simulink model "benchmark_no_taylor"  (Simulink 8.8)
settings: Solver=ode45  StartTime=0.0  StopTime=1000  RelTol=1e-3  EnableMultiTasking=on
undefined symbols (not defined in the file or in its folder): sigbuilder_block
libraries: simulink x11, libturbines x10
(part 2 of 3)

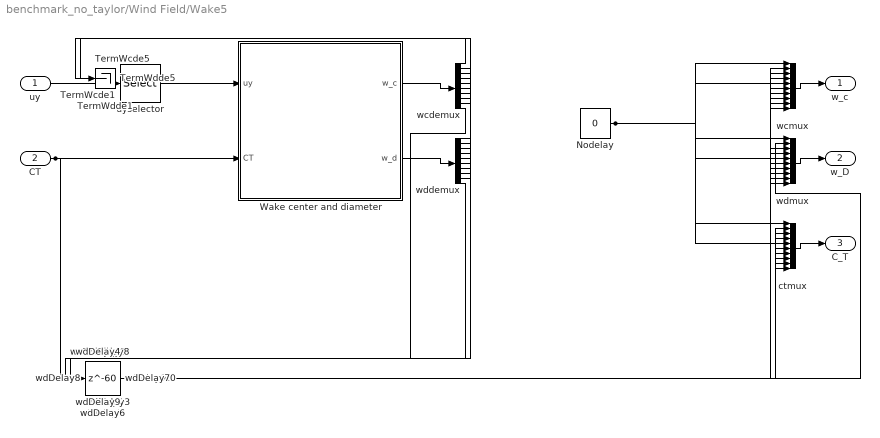
== system benchmark_no_taylor/Wind Field/Wake5 ==
  uy : Inport
  CT : Inport  Port=2
  Nodelay : Constant  Value=0
  TermWcde1 : Terminator
  TermWcde5 : Terminator
  TermWdde1 : Terminator
  TermWdde5 : Terminator
  Wake center and diameter : MATLAB Function  TreatAsAtomicUnit=on
  ctDelay10 : Delay  DelayLength=100  InitialCondition=0  InputProcessing=Inherited  SampleTime=1
  ctDelay2 : Delay  DelayLength=20  InitialCondition=0  InputProcessing=Inherited  SampleTime=1
  ctDelay3 : Delay  DelayLength=60  InitialCondition=0  InputProcessing=Inherited  SampleTime=1
  ctDelay4 : Delay  DelayLength=100  InitialCondition=0  InputProcessing=Inherited  SampleTime=1
  ctDelay6 : Delay  DelayLength=40  InitialCondition=0  InputProcessing=Inherited  SampleTime=1
  ctDelay7 : Delay  DelayLength=80  InitialCondition=0  InputProcessing=Inherited  SampleTime=1
  ctDelay8 : Delay  DelayLength=20  InitialCondition=0  InputProcessing=Inherited  SampleTime=1
  ctDelay9 : Delay  DelayLength=60  InitialCondition=0  InputProcessing=Inherited  SampleTime=1
  ctmux : Mux  Inputs=10
  uyselector : Selector  NumberOfDimensions=2  InputPortWidth=3  IndexOptions=Select all,Index vector (dialog)  Indices=[1 3],p.wt.num  OutputSizes=1,1
  wcDelay10 : Delay  DelayLength=100  InitialCondition=0  InputProcessing=Inherited  SampleTime=1
  wcDelay2 : Delay  DelayLength=20  InitialCondition=0  InputProcessing=Inherited  SampleTime=1
  wcDelay3 : Delay  DelayLength=60  InitialCondition=0  InputProcessing=Inherited  SampleTime=1
  wcDelay4 : Delay  DelayLength=100  InitialCondition=0  InputProcessing=Inherited  SampleTime=1
  wcDelay6 : Delay  DelayLength=40  InitialCondition=0  InputProcessing=Inherited  SampleTime=1
  wcDelay7 : Delay  DelayLength=80  InitialCondition=0  InputProcessing=Inherited  SampleTime=1
  wcDelay8 : Delay  DelayLength=20  InitialCondition=0  InputProcessing=Inherited  SampleTime=1
  wcDelay9 : Delay  DelayLength=60  InitialCondition=0  InputProcessing=Inherited  SampleTime=1
  wcdemux : Demux  Outputs=10
  wcmux : Mux  Inputs=10
  wdDelay10 : Delay  DelayLength=100  InitialCondition=0  InputProcessing=Inherited  SampleTime=1
  wdDelay2 : Delay  DelayLength=20  InitialCondition=0  InputProcessing=Inherited  SampleTime=1
  wdDelay3 : Delay  DelayLength=60  InitialCondition=0  InputProcessing=Inherited  SampleTime=1
  wdDelay4 : Delay  DelayLength=100  InitialCondition=0  InputProcessing=Inherited  SampleTime=1
  wdDelay6 : Delay  DelayLength=40  InitialCondition=0  InputProcessing=Inherited  SampleTime=1
  wdDelay7 : Delay  DelayLength=80  InitialCondition=0  InputProcessing=Inherited  SampleTime=1
  wdDelay8 : Delay  DelayLength=20  InitialCondition=0  InputProcessing=Inherited  SampleTime=1
  wdDelay9 : Delay  DelayLength=60  InitialCondition=0  InputProcessing=Inherited  SampleTime=1
  wddemux : Demux  Outputs=10
  wdmux : Mux  Inputs=10
  w_c : Outport
  w_D : Outport  Port=2
  C_T : Outport  Port=3
  wires:
  ctmux/1 -> C_T/1
  CT/1 -> Wake center and diameter/2(CT), ctDelay2/1, ctDelay3/1, ctDelay4/1, ctDelay6/1, ctDelay7/1, ctDelay8/1, ctDelay9/1, ctDelay10/1
  uy/1 -> uyselector/1
  uyselector/1 -> Wake center and diameter/1(uy)
  Wake center and diameter/1(w_c) -> wcdemux/1
  Wake center and diameter/2(w_d) -> wddemux/1
  wcmux/1 -> w_c/1
  wdmux/1 -> w_D/1
  wddemux/1 -> TermWdde1/1
  wcdemux/1 -> TermWcde1/1
  Nodelay/1 -> wdmux/1, wcmux/1, ctmux/1, wdmux/5, wcmux/5, ctmux/5
  wddemux/2 -> wdDelay2/1
  wdDelay2/1 -> wdmux/2
  wcdemux/2 -> wcDelay2/1
  wcDelay2/1 -> wcmux/2
  ctDelay2/1 -> ctmux/2
  wddemux/3 -> wdDelay3/1
  wdDelay3/1 -> wdmux/3
  wcdemux/3 -> wcDelay3/1
  wcDelay3/1 -> wcmux/3
  ctDelay3/1 -> ctmux/3
  wddemux/4 -> wdDelay4/1
  wdDelay4/1 -> wdmux/4
  wcdemux/4 -> wcDelay4/1
  wcDelay4/1 -> wcmux/4
  ctDelay4/1 -> ctmux/4
  wddemux/5 -> TermWdde5/1
  wcdemux/5 -> TermWcde5/1
  wddemux/6 -> wdDelay6/1
  wdDelay6/1 -> wdmux/6
  wcdemux/6 -> wcDelay6/1
  wcDelay6/1 -> wcmux/6
  ctDelay6/1 -> ctmux/6
  wddemux/7 -> wdDelay7/1
  wdDelay7/1 -> wdmux/7
  wcdemux/7 -> wcDelay7/1
  wcDelay7/1 -> wcmux/7
  ctDelay7/1 -> ctmux/7
  wddemux/8 -> wdDelay8/1
  wdDelay8/1 -> wdmux/8
  wcdemux/8 -> wcDelay8/1
  wcDelay8/1 -> wcmux/8
  ctDelay8/1 -> ctmux/8
  wddemux/9 -> wdDelay9/1
  wdDelay9/1 -> wdmux/9
  wcdemux/9 -> wcDelay9/1
  wcDelay9/1 -> wcmux/9
  ctDelay9/1 -> ctmux/9
  wddemux/10 -> wdDelay10/1
  wdDelay10/1 -> wdmux/10
  wcdemux/10 -> wcDelay10/1
  wcDelay10/1 -> wcmux/10
  ctDelay10/1 -> ctmux/10

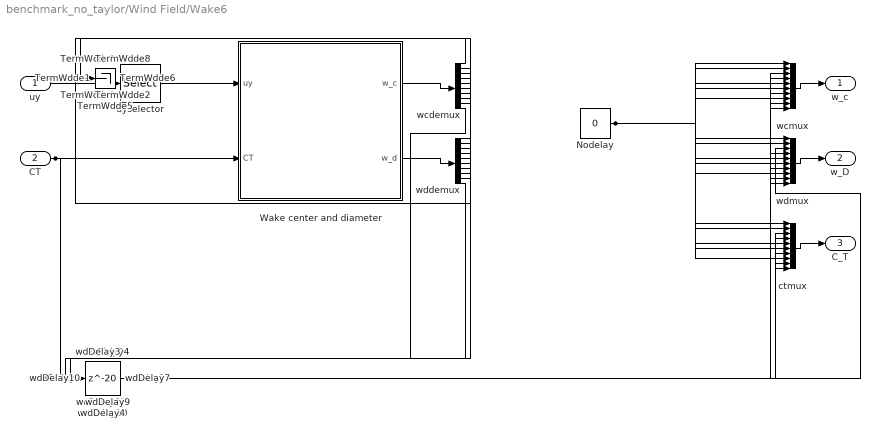
== system benchmark_no_taylor/Wind Field/Wake6 ==
  uy : Inport
  CT : Inport  Port=2
  Nodelay : Constant  Value=0
  TermWcde{1..2,5..6,8} (5 alike) : Terminator
  TermWdde{1..2,5..6,8} (5 alike) : Terminator
  Wake center and diameter : MATLAB Function  TreatAsAtomicUnit=on
  ctDelay10 : Delay  DelayLength=60  InitialCondition=0  InputProcessing=Inherited  SampleTime=1
  ctDelay3 : Delay  DelayLength=20  InitialCondition=0  InputProcessing=Inherited  SampleTime=1
  ctDelay4 : Delay  DelayLength=60  InitialCondition=0  InputProcessing=Inherited  SampleTime=1
  ctDelay7 : Delay  DelayLength=40  InitialCondition=0  InputProcessing=Inherited  SampleTime=1
  ctDelay9 : Delay  DelayLength=20  InitialCondition=0  InputProcessing=Inherited  SampleTime=1
  ctmux : Mux  Inputs=10
  uyselector : Selector  NumberOfDimensions=2  InputPortWidth=3  IndexOptions=Select all,Index vector (dialog)  Indices=[1 3],p.wt.num  OutputSizes=1,1
  wcDelay10 : Delay  DelayLength=60  InitialCondition=0  InputProcessing=Inherited  SampleTime=1
  wcDelay3 : Delay  DelayLength=20  InitialCondition=0  InputProcessing=Inherited  SampleTime=1
  wcDelay4 : Delay  DelayLength=60  InitialCondition=0  InputProcessing=Inherited  SampleTime=1
  wcDelay7 : Delay  DelayLength=40  InitialCondition=0  InputProcessing=Inherited  SampleTime=1
  wcDelay9 : Delay  DelayLength=20  InitialCondition=0  InputProcessing=Inherited  SampleTime=1
  wcdemux : Demux  Outputs=10
  wcmux : Mux  Inputs=10
  wdDelay10 : Delay  DelayLength=60  InitialCondition=0  InputProcessing=Inherited  SampleTime=1
  wdDelay3 : Delay  DelayLength=20  InitialCondition=0  InputProcessing=Inherited  SampleTime=1
  wdDelay4 : Delay  DelayLength=60  InitialCondition=0  InputProcessing=Inherited  SampleTime=1
  wdDelay7 : Delay  DelayLength=40  InitialCondition=0  InputProcessing=Inherited  SampleTime=1
  wdDelay9 : Delay  DelayLength=20  InitialCondition=0  InputProcessing=Inherited  SampleTime=1
  wddemux : Demux  Outputs=10
  wdmux : Mux  Inputs=10
  w_c : Outport
  w_D : Outport  Port=2
  C_T : Outport  Port=3
  wires:
  ctmux/1 -> C_T/1
  CT/1 -> Wake center and diameter/2(CT), ctDelay3/1, ctDelay4/1, ctDelay7/1, ctDelay9/1, ctDelay10/1
  uy/1 -> uyselector/1
  uyselector/1 -> Wake center and diameter/1(uy)
  Wake center and diameter/1(w_c) -> wcdemux/1
  Wake center and diameter/2(w_d) -> wddemux/1
  wcmux/1 -> w_c/1
  wdmux/1 -> w_D/1
  wddemux/1 -> TermWdde1/1
  wcdemux/1 -> TermWcde1/1
  Nodelay/1 -> wdmux/1, wcmux/1, ctmux/1, wdmux/2, wcmux/2, ctmux/2, wdmux/5, wcmux/5, ctmux/5, wdmux/6, wcmux/6, ctmux/6, wdmux/8, wcmux/8, ctmux/8
  wddemux/2 -> TermWdde2/1
  wcdemux/2 -> TermWcde2/1
  wddemux/3 -> wdDelay3/1
  wdDelay3/1 -> wdmux/3
  wcdemux/3 -> wcDelay3/1
  wcDelay3/1 -> wcmux/3
  ctDelay3/1 -> ctmux/3
  wddemux/4 -> wdDelay4/1
  wdDelay4/1 -> wdmux/4
  wcdemux/4 -> wcDelay4/1
  wcDelay4/1 -> wcmux/4
  ctDelay4/1 -> ctmux/4
  wddemux/5 -> TermWdde5/1
  wcdemux/5 -> TermWcde5/1
  wddemux/6 -> TermWdde6/1
  wcdemux/6 -> TermWcde6/1
  wddemux/7 -> wdDelay7/1
  wdDelay7/1 -> wdmux/7
  wcdemux/7 -> wcDelay7/1
  wcDelay7/1 -> wcmux/7
  ctDelay7/1 -> ctmux/7
  wddemux/8 -> TermWdde8/1
  wcdemux/8 -> TermWcde8/1
  wddemux/9 -> wdDelay9/1
  wdDelay9/1 -> wdmux/9
  wcdemux/9 -> wcDelay9/1
  wcDelay9/1 -> wcmux/9
  ctDelay9/1 -> ctmux/9
  wddemux/10 -> wdDelay10/1
  wdDelay10/1 -> wdmux/10
  wcdemux/10 -> wcDelay10/1
  wcDelay10/1 -> wcmux/10
  ctDelay10/1 -> ctmux/10

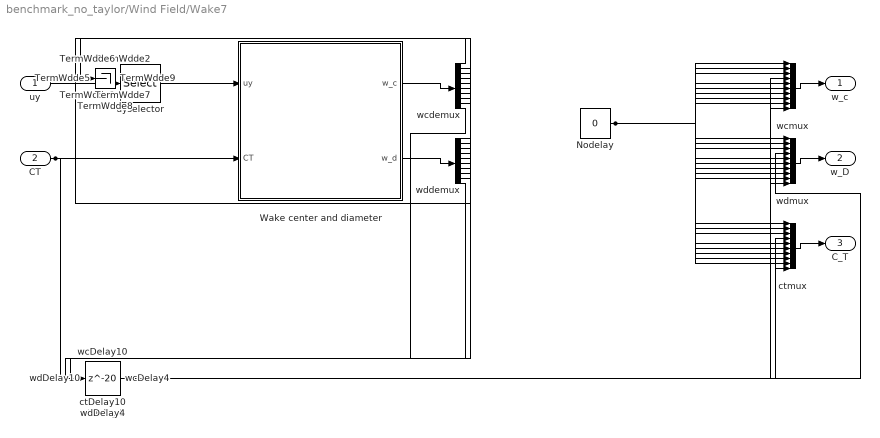
== system benchmark_no_taylor/Wind Field/Wake7 ==
  uy : Inport
  CT : Inport  Port=2
  Nodelay : Constant  Value=0
  TermWcde{1..3,5..9} (8 alike) : Terminator
  TermWdde{1..3,5..9} (8 alike) : Terminator
  Wake center and diameter : MATLAB Function  TreatAsAtomicUnit=on
  ctDelay10 : Delay  DelayLength=20  InitialCondition=0  InputProcessing=Inherited  SampleTime=1
  ctDelay4 : Delay  DelayLength=20  InitialCondition=0  InputProcessing=Inherited  SampleTime=1
  ctmux : Mux  Inputs=10
  uyselector : Selector  NumberOfDimensions=2  InputPortWidth=3  IndexOptions=Select all,Index vector (dialog)  Indices=[1 3],p.wt.num  OutputSizes=1,1
  wcDelay10 : Delay  DelayLength=20  InitialCondition=0  InputProcessing=Inherited  SampleTime=1
  wcDelay4 : Delay  DelayLength=20  InitialCondition=0  InputProcessing=Inherited  SampleTime=1
  wcdemux : Demux  Outputs=10
  wcmux : Mux  Inputs=10
  wdDelay10 : Delay  DelayLength=20  InitialCondition=0  InputProcessing=Inherited  SampleTime=1
  wdDelay4 : Delay  DelayLength=20  InitialCondition=0  InputProcessing=Inherited  SampleTime=1
  wddemux : Demux  Outputs=10
  wdmux : Mux  Inputs=10
  w_c : Outport
  w_D : Outport  Port=2
  C_T : Outport  Port=3
  wires:
  ctmux/1 -> C_T/1
  CT/1 -> Wake center and diameter/2(CT), ctDelay4/1, ctDelay10/1
  uy/1 -> uyselector/1
  uyselector/1 -> Wake center and diameter/1(uy)
  Wake center and diameter/1(w_c) -> wcdemux/1
  Wake center and diameter/2(w_d) -> wddemux/1
  wcmux/1 -> w_c/1
  wdmux/1 -> w_D/1
  wddemux/1 -> TermWdde1/1
  wcdemux/1 -> TermWcde1/1
  Nodelay/1 -> wdmux/1, wcmux/1, ctmux/1, wdmux/2, wcmux/2, ctmux/2, wdmux/3, wcmux/3, ctmux/3, wdmux/5, wcmux/5, ctmux/5, wdmux/6, wcmux/6, ctmux/6, wdmux/7, wcmux/7, ctmux/7, wdmux/8, wcmux/8, ctmux/8, wdmux/9, wcmux/9, ctmux/9
  wddemux/2 -> TermWdde2/1
  wcdemux/2 -> TermWcde2/1
  wddemux/3 -> TermWdde3/1
  wcdemux/3 -> TermWcde3/1
  wddemux/4 -> wdDelay4/1
  wdDelay4/1 -> wdmux/4
  wcdemux/4 -> wcDelay4/1
  wcDelay4/1 -> wcmux/4
  ctDelay4/1 -> ctmux/4
  wddemux/5 -> TermWdde5/1
  wcdemux/5 -> TermWcde5/1
  wddemux/6 -> TermWdde6/1
  wcdemux/6 -> TermWcde6/1
  wddemux/7 -> TermWdde7/1
  wcdemux/7 -> TermWcde7/1
  wddemux/8 -> TermWdde8/1
  wcdemux/8 -> TermWcde8/1
  wddemux/9 -> TermWdde9/1
  wcdemux/9 -> TermWcde9/1
  wddemux/10 -> wdDelay10/1
  wdDelay10/1 -> wdmux/10
  wcdemux/10 -> wcDelay10/1
  wcDelay10/1 -> wcmux/10
  ctDelay10/1 -> ctmux/10

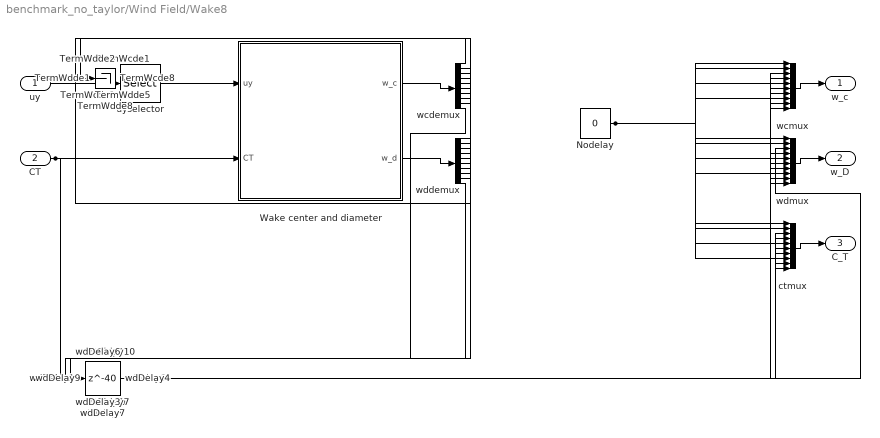
== system benchmark_no_taylor/Wind Field/Wake8 ==
  uy : Inport
  CT : Inport  Port=2
  Nodelay : Constant  Value=0
  TermWcde{1..2,5,8} (4 alike) : Terminator
  TermWdde{1..2,5,8} (4 alike) : Terminator
  Wake center and diameter : MATLAB Function  TreatAsAtomicUnit=on
  ctDelay10 : Delay  DelayLength=80  InitialCondition=0  InputProcessing=Inherited  SampleTime=1
  ctDelay3 : Delay  DelayLength=40  InitialCondition=0  InputProcessing=Inherited  SampleTime=1
  ctDelay4 : Delay  DelayLength=80  InitialCondition=0  InputProcessing=Inherited  SampleTime=1
  ctDelay6 : Delay  DelayLength=20  InitialCondition=0  InputProcessing=Inherited  SampleTime=1
  ctDelay7 : Delay  DelayLength=60  InitialCondition=0  InputProcessing=Inherited  SampleTime=1
  ctDelay9 : Delay  DelayLength=40  InitialCondition=0  InputProcessing=Inherited  SampleTime=1
  ctmux : Mux  Inputs=10
  uyselector : Selector  NumberOfDimensions=2  InputPortWidth=3  IndexOptions=Select all,Index vector (dialog)  Indices=[1 3],p.wt.num  OutputSizes=1,1
  wcDelay10 : Delay  DelayLength=80  InitialCondition=0  InputProcessing=Inherited  SampleTime=1
  wcDelay3 : Delay  DelayLength=40  InitialCondition=0  InputProcessing=Inherited  SampleTime=1
  wcDelay4 : Delay  DelayLength=80  InitialCondition=0  InputProcessing=Inherited  SampleTime=1
  wcDelay6 : Delay  DelayLength=20  InitialCondition=0  InputProcessing=Inherited  SampleTime=1
  wcDelay7 : Delay  DelayLength=60  InitialCondition=0  InputProcessing=Inherited  SampleTime=1
  wcDelay9 : Delay  DelayLength=40  InitialCondition=0  InputProcessing=Inherited  SampleTime=1
  wcdemux : Demux  Outputs=10
  wcmux : Mux  Inputs=10
  wdDelay10 : Delay  DelayLength=80  InitialCondition=0  InputProcessing=Inherited  SampleTime=1
  wdDelay3 : Delay  DelayLength=40  InitialCondition=0  InputProcessing=Inherited  SampleTime=1
  wdDelay4 : Delay  DelayLength=80  InitialCondition=0  InputProcessing=Inherited  SampleTime=1
  wdDelay6 : Delay  DelayLength=20  InitialCondition=0  InputProcessing=Inherited  SampleTime=1
  wdDelay7 : Delay  DelayLength=60  InitialCondition=0  InputProcessing=Inherited  SampleTime=1
  wdDelay9 : Delay  DelayLength=40  InitialCondition=0  InputProcessing=Inherited  SampleTime=1
  wddemux : Demux  Outputs=10
  wdmux : Mux  Inputs=10
  w_c : Outport
  w_D : Outport  Port=2
  C_T : Outport  Port=3
  wires:
  ctmux/1 -> C_T/1
  CT/1 -> Wake center and diameter/2(CT), ctDelay3/1, ctDelay4/1, ctDelay6/1, ctDelay7/1, ctDelay9/1, ctDelay10/1
  uy/1 -> uyselector/1
  uyselector/1 -> Wake center and diameter/1(uy)
  Wake center and diameter/1(w_c) -> wcdemux/1
  Wake center and diameter/2(w_d) -> wddemux/1
  wcmux/1 -> w_c/1
  wdmux/1 -> w_D/1
  wddemux/1 -> TermWdde1/1
  wcdemux/1 -> TermWcde1/1
  Nodelay/1 -> wdmux/1, wcmux/1, ctmux/1, wdmux/2, wcmux/2, ctmux/2, wdmux/5, wcmux/5, ctmux/5, wdmux/8, wcmux/8, ctmux/8
  wddemux/2 -> TermWdde2/1
  wcdemux/2 -> TermWcde2/1
  wddemux/3 -> wdDelay3/1
  wdDelay3/1 -> wdmux/3
  wcdemux/3 -> wcDelay3/1
  wcDelay3/1 -> wcmux/3
  ctDelay3/1 -> ctmux/3
  wddemux/4 -> wdDelay4/1
  wdDelay4/1 -> wdmux/4
  wcdemux/4 -> wcDelay4/1
  wcDelay4/1 -> wcmux/4
  ctDelay4/1 -> ctmux/4
  wddemux/5 -> TermWdde5/1
  wcdemux/5 -> TermWcde5/1
  wddemux/6 -> wdDelay6/1
  wdDelay6/1 -> wdmux/6
  wcdemux/6 -> wcDelay6/1
  wcDelay6/1 -> wcmux/6
  ctDelay6/1 -> ctmux/6
  wddemux/7 -> wdDelay7/1
  wdDelay7/1 -> wdmux/7
  wcdemux/7 -> wcDelay7/1
  wcDelay7/1 -> wcmux/7
  ctDelay7/1 -> ctmux/7
  wddemux/8 -> TermWdde8/1
  wcdemux/8 -> TermWcde8/1
  wddemux/9 -> wdDelay9/1
  wdDelay9/1 -> wdmux/9
  wcdemux/9 -> wcDelay9/1
  wcDelay9/1 -> wcmux/9
  ctDelay9/1 -> ctmux/9
  wddemux/10 -> wdDelay10/1
  wdDelay10/1 -> wdmux/10
  wcdemux/10 -> wcDelay10/1
  wcDelay10/1 -> wcmux/10
  ctDelay10/1 -> ctmux/10

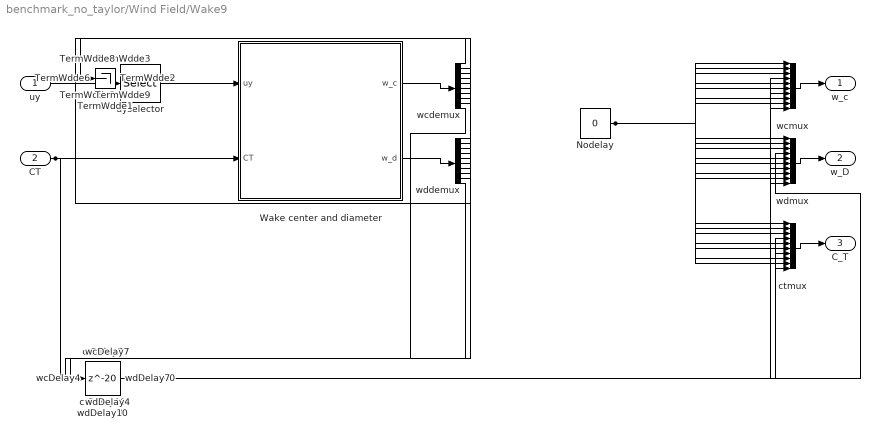
== system benchmark_no_taylor/Wind Field/Wake9 ==
  uy : Inport
  CT : Inport  Port=2
  Nodelay : Constant  Value=0
  TermWcde{1..3,5..6,8..9} (7 alike) : Terminator
  TermWdde{1..3,5..6,8..9} (7 alike) : Terminator
  Wake center and diameter : MATLAB Function  TreatAsAtomicUnit=on
  ctDelay10 : Delay  DelayLength=40  InitialCondition=0  InputProcessing=Inherited  SampleTime=1
  ctDelay4 : Delay  DelayLength=40  InitialCondition=0  InputProcessing=Inherited  SampleTime=1
  ctDelay7 : Delay  DelayLength=20  InitialCondition=0  InputProcessing=Inherited  SampleTime=1
  ctmux : Mux  Inputs=10
  uyselector : Selector  NumberOfDimensions=2  InputPortWidth=3  IndexOptions=Select all,Index vector (dialog)  Indices=[1 3],p.wt.num  OutputSizes=1,1
  wcDelay10 : Delay  DelayLength=40  InitialCondition=0  InputProcessing=Inherited  SampleTime=1
  wcDelay4 : Delay  DelayLength=40  InitialCondition=0  InputProcessing=Inherited  SampleTime=1
  wcDelay7 : Delay  DelayLength=20  InitialCondition=0  InputProcessing=Inherited  SampleTime=1
  wcdemux : Demux  Outputs=10
  wcmux : Mux  Inputs=10
  wdDelay10 : Delay  DelayLength=40  InitialCondition=0  InputProcessing=Inherited  SampleTime=1
  wdDelay4 : Delay  DelayLength=40  InitialCondition=0  InputProcessing=Inherited  SampleTime=1
  wdDelay7 : Delay  DelayLength=20  InitialCondition=0  InputProcessing=Inherited  SampleTime=1
  wddemux : Demux  Outputs=10
  wdmux : Mux  Inputs=10
  w_c : Outport
  w_D : Outport  Port=2
  C_T : Outport  Port=3
  wires:
  ctmux/1 -> C_T/1
  CT/1 -> Wake center and diameter/2(CT), ctDelay4/1, ctDelay7/1, ctDelay10/1
  uy/1 -> uyselector/1
  uyselector/1 -> Wake center and diameter/1(uy)
  Wake center and diameter/1(w_c) -> wcdemux/1
  Wake center and diameter/2(w_d) -> wddemux/1
  wcmux/1 -> w_c/1
  wdmux/1 -> w_D/1
  wddemux/1 -> TermWdde1/1
  wcdemux/1 -> TermWcde1/1
  Nodelay/1 -> wdmux/1, wcmux/1, ctmux/1, wdmux/2, wcmux/2, ctmux/2, wdmux/3, wcmux/3, ctmux/3, wdmux/5, wcmux/5, ctmux/5, wdmux/6, wcmux/6, ctmux/6, wdmux/8, wcmux/8, ctmux/8, wdmux/9, wcmux/9, ctmux/9
  wddemux/2 -> TermWdde2/1
  wcdemux/2 -> TermWcde2/1
  wddemux/3 -> TermWdde3/1
  wcdemux/3 -> TermWcde3/1
  wddemux/4 -> wdDelay4/1
  wdDelay4/1 -> wdmux/4
  wcdemux/4 -> wcDelay4/1
  wcDelay4/1 -> wcmux/4
  ctDelay4/1 -> ctmux/4
  wddemux/5 -> TermWdde5/1
  wcdemux/5 -> TermWcde5/1
  wddemux/6 -> TermWdde6/1
  wcdemux/6 -> TermWcde6/1
  wddemux/7 -> wdDelay7/1
  wdDelay7/1 -> wdmux/7
  wcdemux/7 -> wcDelay7/1
  wcDelay7/1 -> wcmux/7
  ctDelay7/1 -> ctmux/7
  wddemux/8 -> TermWdde8/1
  wcdemux/8 -> TermWcde8/1
  wddemux/9 -> TermWdde9/1
  wcdemux/9 -> TermWcde9/1
  wddemux/10 -> wdDelay10/1
  wdDelay10/1 -> wdmux/10
  wcdemux/10 -> wcDelay10/1
  wcDelay10/1 -> wcmux/10
  ctDelay10/1 -> ctmux/10

== system benchmark_no_taylor/Wind Field/Wind Speed1 ==
  def : Inport
  Counter Free-Running : simulink/Sources/Counter Free-Running  NumBits=32  tsamp=p.ts
  Selector1 : Selector  NumberOfDimensions=2  IndexMode=Zero-based  InputPortWidth=3  IndexOptions=Select all,Index vector (port)  Indices=[1 3],1  OutputSizes=1,1
  Signal Conversion : SignalConversion  ConversionOutput=Contiguous copy  OverrideOpt=off
  Signal Conversion1 : SignalConversion  ConversionOutput=Contiguous copy  OverrideOpt=off
  Ux_full : Constant  Value=p.wind'
  Wind speed : MATLAB Function  TreatAsAtomicUnit=on
  v_rot : Outport
  v_nac : Outport  Port=2
  wires:
  def/1 -> Wind speed/2(def)
  Wind speed/2(upoint) -> Signal Conversion1/1
  Wind speed/1(uavg) -> Signal Conversion/1
  Counter Free-Running/1 -> Selector1/2
  Ux_full/1 -> Selector1/1
  Selector1/1 -> Wind speed/1(ux)
  Signal Conversion/1 -> v_rot/1
  Signal Conversion1/1 -> v_nac/1

== system benchmark_no_taylor/Wind Field/Wind Speed10 ==
  def : Inport
  Counter Free-Running : simulink/Sources/Counter Free-Running  NumBits=32  tsamp=p.ts
  Selector1 : Selector  NumberOfDimensions=2  IndexMode=Zero-based  InputPortWidth=3  IndexOptions=Select all,Index vector (port)  Indices=[1 3],1  OutputSizes=1,1
  Signal Conversion : SignalConversion  ConversionOutput=Contiguous copy  OverrideOpt=off
  Signal Conversion1 : SignalConversion  ConversionOutput=Contiguous copy  OverrideOpt=off
  Ux_full : Constant  Value=p.wind'
  Wind speed : MATLAB Function  TreatAsAtomicUnit=on
  v_rot : Outport
  v_nac : Outport  Port=2
  wires:
  def/1 -> Wind speed/2(def)
  Wind speed/2(upoint) -> Signal Conversion1/1
  Wind speed/1(uavg) -> Signal Conversion/1
  Counter Free-Running/1 -> Selector1/2
  Ux_full/1 -> Selector1/1
  Selector1/1 -> Wind speed/1(ux)
  Signal Conversion/1 -> v_rot/1
  Signal Conversion1/1 -> v_nac/1

== system benchmark_no_taylor/Wind Field/Wind Speed2 ==
  def : Inport
  Counter Free-Running : simulink/Sources/Counter Free-Running  NumBits=32  tsamp=p.ts
  Selector1 : Selector  NumberOfDimensions=2  IndexMode=Zero-based  InputPortWidth=3  IndexOptions=Select all,Index vector (port)  Indices=[1 3],1  OutputSizes=1,1
  Signal Conversion : SignalConversion  ConversionOutput=Contiguous copy  OverrideOpt=off
  Signal Conversion1 : SignalConversion  ConversionOutput=Contiguous copy  OverrideOpt=off
  Ux_full : Constant  Value=p.wind'
  Wind speed : MATLAB Function  TreatAsAtomicUnit=on
  v_rot : Outport
  v_nac : Outport  Port=2
  wires:
  def/1 -> Wind speed/2(def)
  Wind speed/2(upoint) -> Signal Conversion1/1
  Wind speed/1(uavg) -> Signal Conversion/1
  Counter Free-Running/1 -> Selector1/2
  Ux_full/1 -> Selector1/1
  Selector1/1 -> Wind speed/1(ux)
  Signal Conversion/1 -> v_rot/1
  Signal Conversion1/1 -> v_nac/1

== system benchmark_no_taylor/Wind Field/Wind Speed3 ==
  def : Inport
  Counter Free-Running : simulink/Sources/Counter Free-Running  NumBits=32  tsamp=p.ts
  Selector1 : Selector  NumberOfDimensions=2  IndexMode=Zero-based  InputPortWidth=3  IndexOptions=Select all,Index vector (port)  Indices=[1 3],1  OutputSizes=1,1
  Signal Conversion : SignalConversion  ConversionOutput=Contiguous copy  OverrideOpt=off
  Signal Conversion1 : SignalConversion  ConversionOutput=Contiguous copy  OverrideOpt=off
  Ux_full : Constant  Value=p.wind'
  Wind speed : MATLAB Function  TreatAsAtomicUnit=on
  v_rot : Outport
  v_nac : Outport  Port=2
  wires:
  def/1 -> Wind speed/2(def)
  Wind speed/2(upoint) -> Signal Conversion1/1
  Wind speed/1(uavg) -> Signal Conversion/1
  Counter Free-Running/1 -> Selector1/2
  Ux_full/1 -> Selector1/1
  Selector1/1 -> Wind speed/1(ux)
  Signal Conversion/1 -> v_rot/1
  Signal Conversion1/1 -> v_nac/1

== system benchmark_no_taylor/Wind Field/Wind Speed4 ==
  def : Inport
  Counter Free-Running : simulink/Sources/Counter Free-Running  NumBits=32  tsamp=p.ts
  Selector1 : Selector  NumberOfDimensions=2  IndexMode=Zero-based  InputPortWidth=3  IndexOptions=Select all,Index vector (port)  Indices=[1 3],1  OutputSizes=1,1
  Signal Conversion : SignalConversion  ConversionOutput=Contiguous copy  OverrideOpt=off
  Signal Conversion1 : SignalConversion  ConversionOutput=Contiguous copy  OverrideOpt=off
  Ux_full : Constant  Value=p.wind'
  Wind speed : MATLAB Function  TreatAsAtomicUnit=on
  v_rot : Outport
  v_nac : Outport  Port=2
  wires:
  def/1 -> Wind speed/2(def)
  Wind speed/2(upoint) -> Signal Conversion1/1
  Wind speed/1(uavg) -> Signal Conversion/1
  Counter Free-Running/1 -> Selector1/2
  Ux_full/1 -> Selector1/1
  Selector1/1 -> Wind speed/1(ux)
  Signal Conversion/1 -> v_rot/1
  Signal Conversion1/1 -> v_nac/1

== system benchmark_no_taylor/Wind Field/Wind Speed5 ==
  def : Inport
  Counter Free-Running : simulink/Sources/Counter Free-Running  NumBits=32  tsamp=p.ts
  Selector1 : Selector  NumberOfDimensions=2  IndexMode=Zero-based  InputPortWidth=3  IndexOptions=Select all,Index vector (port)  Indices=[1 3],1  OutputSizes=1,1
  Signal Conversion : SignalConversion  ConversionOutput=Contiguous copy  OverrideOpt=off
  Signal Conversion1 : SignalConversion  ConversionOutput=Contiguous copy  OverrideOpt=off
  Ux_full : Constant  Value=p.wind'
  Wind speed : MATLAB Function  TreatAsAtomicUnit=on
  v_rot : Outport
  v_nac : Outport  Port=2
  wires:
  def/1 -> Wind speed/2(def)
  Wind speed/2(upoint) -> Signal Conversion1/1
  Wind speed/1(uavg) -> Signal Conversion/1
  Counter Free-Running/1 -> Selector1/2
  Ux_full/1 -> Selector1/1
  Selector1/1 -> Wind speed/1(ux)
  Signal Conversion/1 -> v_rot/1
  Signal Conversion1/1 -> v_nac/1

== system benchmark_no_taylor/Wind Field/Wind Speed6 ==
  def : Inport
  Counter Free-Running : simulink/Sources/Counter Free-Running  NumBits=32  tsamp=p.ts
  Selector1 : Selector  NumberOfDimensions=2  IndexMode=Zero-based  InputPortWidth=3  IndexOptions=Select all,Index vector (port)  Indices=[1 3],1  OutputSizes=1,1
  Signal Conversion : SignalConversion  ConversionOutput=Contiguous copy  OverrideOpt=off
  Signal Conversion1 : SignalConversion  ConversionOutput=Contiguous copy  OverrideOpt=off
  Ux_full : Constant  Value=p.wind'
  Wind speed : MATLAB Function  TreatAsAtomicUnit=on
  v_rot : Outport
  v_nac : Outport  Port=2
  wires:
  def/1 -> Wind speed/2(def)
  Wind speed/2(upoint) -> Signal Conversion1/1
  Wind speed/1(uavg) -> Signal Conversion/1
  Counter Free-Running/1 -> Selector1/2
  Ux_full/1 -> Selector1/1
  Selector1/1 -> Wind speed/1(ux)
  Signal Conversion/1 -> v_rot/1
  Signal Conversion1/1 -> v_nac/1

== system benchmark_no_taylor/Wind Field/Wind Speed7 ==
  def : Inport
  Counter Free-Running : simulink/Sources/Counter Free-Running  NumBits=32  tsamp=p.ts
  Selector1 : Selector  NumberOfDimensions=2  IndexMode=Zero-based  InputPortWidth=3  IndexOptions=Select all,Index vector (port)  Indices=[1 3],1  OutputSizes=1,1
  Signal Conversion : SignalConversion  ConversionOutput=Contiguous copy  OverrideOpt=off
  Signal Conversion1 : SignalConversion  ConversionOutput=Contiguous copy  OverrideOpt=off
  Ux_full : Constant  Value=p.wind'
  Wind speed : MATLAB Function  TreatAsAtomicUnit=on
  v_rot : Outport
  v_nac : Outport  Port=2
  wires:
  def/1 -> Wind speed/2(def)
  Wind speed/2(upoint) -> Signal Conversion1/1
  Wind speed/1(uavg) -> Signal Conversion/1
  Counter Free-Running/1 -> Selector1/2
  Ux_full/1 -> Selector1/1
  Selector1/1 -> Wind speed/1(ux)
  Signal Conversion/1 -> v_rot/1
  Signal Conversion1/1 -> v_nac/1

== system benchmark_no_taylor/Wind Field/Wind Speed8 ==
  def : Inport
  Counter Free-Running : simulink/Sources/Counter Free-Running  NumBits=32  tsamp=p.ts
  Selector1 : Selector  NumberOfDimensions=2  IndexMode=Zero-based  InputPortWidth=3  IndexOptions=Select all,Index vector (port)  Indices=[1 3],1  OutputSizes=1,1
  Signal Conversion : SignalConversion  ConversionOutput=Contiguous copy  OverrideOpt=off
  Signal Conversion1 : SignalConversion  ConversionOutput=Contiguous copy  OverrideOpt=off
  Ux_full : Constant  Value=p.wind'
  Wind speed : MATLAB Function  TreatAsAtomicUnit=on
  v_rot : Outport
  v_nac : Outport  Port=2
  wires:
  def/1 -> Wind speed/2(def)
  Wind speed/2(upoint) -> Signal Conversion1/1
  Wind speed/1(uavg) -> Signal Conversion/1
  Counter Free-Running/1 -> Selector1/2
  Ux_full/1 -> Selector1/1
  Selector1/1 -> Wind speed/1(ux)
  Signal Conversion/1 -> v_rot/1
  Signal Conversion1/1 -> v_nac/1

== system benchmark_no_taylor/Wind Field/Wind Speed9 ==
  def : Inport
  Counter Free-Running : simulink/Sources/Counter Free-Running  NumBits=32  tsamp=p.ts
  Selector1 : Selector  NumberOfDimensions=2  IndexMode=Zero-based  InputPortWidth=3  IndexOptions=Select all,Index vector (port)  Indices=[1 3],1  OutputSizes=1,1
  Signal Conversion : SignalConversion  ConversionOutput=Contiguous copy  OverrideOpt=off
  Signal Conversion1 : SignalConversion  ConversionOutput=Contiguous copy  OverrideOpt=off
  Ux_full : Constant  Value=p.wind'
  Wind speed : MATLAB Function  TreatAsAtomicUnit=on
  v_rot : Outport
  v_nac : Outport  Port=2
  wires:
  def/1 -> Wind speed/2(def)
  Wind speed/2(upoint) -> Signal Conversion1/1
  Wind speed/1(uavg) -> Signal Conversion/1
  Counter Free-Running/1 -> Selector1/2
  Ux_full/1 -> Selector1/1
  Selector1/1 -> Wind speed/1(ux)
  Signal Conversion/1 -> v_rot/1
  Signal Conversion1/1 -> v_nac/1

== matlab function Farm Control/control ==
  data: v_nac input double; P_demand input double; parm parameter double; P_ref output double; P_avail output double
function [P_ref,P_avail]  = Farm_Controller(v_nac,P_demand,parm)
%
% Farm_Controller estimates the available power at the farm and distributes the 
% power references proportional to the available power at each wind turbine.
%
% [P_ref,P_avail_total]  = Farm_Controller(v_nac,P_demand)
%
% vn : the wind speed at wind turbine number n.
%
% P_demand : The demanded power by the operator.
%
% P_ref : The power reference for the wind turbines.
%
% P_avail : Available power at the farm level
%
%
% Version 1: 2009-04-10 sms
% Version 2: 2009-12-04 grunnet
% By : S. Mohsen N. Soltani & Jacob D. Grunnet
% Aalborg University, Dept. of Electronic Systems, Section of Automation
% and Control
% E-mail: sms@es.aau.dk
% E-mail: grunnet@es.aau.dk

rho=parm.rho; %air dencity
R=parm.radius; %rotor radius
rated=parm.rated; %Rated power
N=parm.N; %Number of turbines
Cp=parm.Cp; % Max cp of the turbines

P_a=zeros(N,1);
P_ref=zeros(N,1);

% Compute available power at each turbine
for i=1:N
    %P_a=1/2*pi*r^2*Cp*v^3
    P_a(i)=min(rated(i),(pi/2)*rho*R(i)^2*v_nac(i)^3*Cp(i));
end

%Compute total available power
P_avail=sum(P_a);

%Distribute power according to availibility
for i=1:N
    P_ref(i)=max(0,min(rated(i),P_demand*P_a(i)/P_avail));
end

== matlab function Network Operator/Network Operator ==
  data: P_avail input double; settings input double; f input double; P_demand output double; P_demand0 input double; parm parameter double
function P_demand = TSO(P_avail,settings,f,P_demand0,parm)
%
% TSO provides the demanded power to the wind farm controller.
%
% P_avail : Aavailable power at the farm level.
%
% mode : The operation mode.
%
% settings : The settings corresponding to the operational mode
%
% f : Frequency of the network
%
% P_deamnd0 : The last update of the deamded power
%
% Ts : Sample Time
%
% Time-stamp: <2009-04-10 sms>
% Version 1: 2009-04-10 sms 
% By : S. Mohsen N. Soltani
% Aalborg University, Dept. of Electronic Systems, Section of Automation
% and Control
% E-mail: sms@es.aau.dk
% 
 P=0;
 switch(parm.mode)
     case 1 %Absolute active power control
        P=settings(1);
%       % type='Full power';
     case 2 %Delta mode
        P=P_avail-settings(1);
%       % type='Delta mode';
     case 3 %Balance
        P=settings(1);
%       % type='Balance';
     case 4 %Rate limiter
        P=min(P_avail,settings(1)*parm.Ts+P_demand0);
%       % type='Rate limiter';
     case 5 %Frequency Control
        deadb=settings(1);
        contb=settings(2);
        maxp=settings(3);
        minp=settings(4);
        f=f-settings(5); % To provide Delta f
        if ( f<= deadb && f>=-deadb)
            P=0.5*(maxp+minp);
        elseif f>= contb
            P=minp;
        elseif f<= -contb
            P=maxp;
        elseif ( f>deadb && f<contb)
            P=0.5*(maxp+minp)-0.5*(maxp-minp)*(f-deadb)/(contb-deadb);
        elseif ( f<-deadb && f>-contb)
            P=0.5*(maxp+minp)-0.5*(maxp-minp)*(f+deadb)/(contb-deadb);
        end
%       % type='Frequency Control';
     otherwise %Unknown
        P=P_avail;
%       % type='Unknown Mode';
 end
%        

P_demand=max(0,P);

== matlab function Wind Field/Deficit ==
  data: w_c input double; w_d input double; ct_dw input double; p parameter double; def output double
function def  = deficit(w_c,w_d,ct_dw,p) %#eml
% w_c   is the vector of wake centers from the other turbins at the x position of the turbine
% w_d   is the vector of wake diameters from the other turbins at the x position of the turbine
% ct_dw is the vector of thrust coefficients from the other turbins at the x position of the turbine
% p     is a structure containing farm layout and tubine rotor sizes  

wake_from = zeros(length(p.farm.x),length(p.farm.x));
dist_i = zeros(length(p.farm.x),1);
def = ones(length(p.farm.x),1);
if ct_dw(size(ct_dw,1),1) ~= 0 % only calculate deficit if wind has reached end of farm
    [~, dist_i] = sort(p.farm.x);

    %This for-loop make a "tree" of which turbines are in the wake
    for i=1:length(p.farm.x)
        turb_i = dist_i(i);
        for k = 1:length(p.farm.x)
            turb_k = dist_i(k);
            temp = p.farm.y(turb_k)-w_c(turb_k,turb_i);
            %if turb_k is in turb_i's wake it is included in the "tree"
            if(temp > -w_d(turb_k,turb_i) && temp < w_d(turb_k,turb_i))  
                wake_from(i,k) = 1; %
            end
        end
    end
    wake_from_2 = wake_from; %copy original wake tree for later use

    for i=1:length(p.farm.x)
        % remove parents from tree if children is in wake = use iterative scheme
        pp = find(wake_from(:,i)==1);
        c = find(wake_from(i,:)==1);
        wake_from(pp,c) = 0;
        switch length(pp)
            case 0 % if no wake the deficit is one
                def(i) = 1;
            case 1 % if one wake then calculate deficit using iterative scheme
                wake_upstream = find(wake_from_2(:,i)==1); %find the upstream turbines
                wd = w_d(dist_i(i),dist_i(min(wake_upstream))); %find wake diameter for "most" upstream turbine
                tmp_ct = ct_dw(dist_i(i),dist_i(max(wake_upstream))); % find CT for closest upstream turbine
                tmp_rotor_D = 2*p.rotor.r(dist_i(max(wake_upstream))); % precalculate rotor diameter for closest upstream turbine
                if(length(wake_upstream) == 1) % if only one upstream turbine use "normal" deficit calculation
                    def(i) = 1-0.5*tmp_rotor_D^2/wd^2*tmp_ct;
                else % else use iterative scheme : "Analytical Modelling of Wind Speed Deficit in Large Offshore Wind Farms" eq:17
                    tmp_def = def(max(wake_upstream)); %find deficit at closest upstream turbine
                    tmp_D = w_d(dist_i(max(wake_upstream)),dist_i(min(wake_upstream))); %find diameter of wake at closest upstream turbine
                    def(i) = 1-(tmp_D^2/wd^2*(1-tmp_def)+0.5*tmp_rotor_D^2/wd^2*tmp_ct*tmp_def); %calculate deficit
                end
            otherwise % if more than wakes meet (overlap but not in row) merge according to area
                %error = 1 % uncomment to see when wakes are merged from other rows
                wake_upstream = find(wake_from_2(:,i)==1);
                wd = w_d(dist_i(i),dist_i(min(wake_upstream)));
                tmp_def = ones(length(pp),1);
                tmp_D = ones(length(pp),1);
                k = 1;
                for l=length(pp) %calculate deficit from each upstream turbine
                    j = pp(l);
                    tmp_ct = ct_dw(dist_i(i),dist_i(j));
                    tmp_def_up = def(dist_i(j));
                    tmp_rotor_D = 2*p.rotor.r(dist_i(max(wake_upstream)));
                    tmp_D(k) = w_d(dist_i(j),dist_i(min(wake_upstream)));
                    tmp_def(k) = 1-(tmp_D(k)^2/wd^2*(1-tmp_def_up)+0.5*tmp_rotor_D^2/wd^2*tmp_ct*tmp_def_up);
                    k = k+1;
                end
                def(i) = sum(tmp_D.^2.*tmp_def)/sum(tmp_D.^2); %combine deficit according to area
        end
    end
    def(dist_i) = def; % Reshuffle deficits such that turbine number is correct
end

== matlab function Wind Field/Wake1/Wake center and diameter ==
  data: uy input double; CT input double; p parameter double; w_c output double; w_d output double
function [w_c,w_d]=wake(uy,CT,p)
    % Todo: Change length(uy)*grid_size to farm size?
    %       Change such that parm is passed directly

    w_c=zeros(length(p.farm.x),1);
    w_d=zeros(length(p.farm.x),1);
    if CT < 1
        beta_w = (1+sqrt(1-CT))/(2*sqrt(1-CT));
    else
        beta_w = 1;
    end
    %beta_w = (1+sqrt(1-CT))/(2*sqrt(1-CT));
    %Find wake center and deficit for all other turbines
    for i=1:length(p.farm.x)
        delta_dist = p.farm.x(i)-p.wt.x;
        if(delta_dist>0) %Is turbine P downwind?
            w_d(i)= (beta_w^(p.k/2) +p.alpha*delta_dist/(2*p.wt.rotor.r))^(1/p.k)*2*p.wt.rotor.r;
            w_c(i)=wakecenter(uy,p.farm.x(i),p);%max(min(wakecenter(uy,p.farm.x(i),p),p.grid.ysize),1);
            
        else
            w_c(i)=p.wt.y;
            w_d(i)=0;
        end
    end
end


function wc=wakecenter(uy,px,p)
    
    %computer number of grid points to px
    it=ceil((px-p.wt.x)/p.grid.size);
    
    wc=p.wt.y; %wake center starts at turbine position
    
    for i=1:it %Compute the meandering for each grid point
        uysum=0;
        %compute the grid number for low meandering edge
        m_min=max(floor((wc-p.wt.meand.r(i))/p.grid.size)+1,1); 
        %compute the grid number for the high meandering edge
        m_max=min(ceil((wc+p.wt.meand.r(i))/p.grid.size)+1,length(uy)); 
        
        %average transversal velocity over the wake area
        for j=m_min:m_max
            uysum=uysum+uy(j);
        end
        uyavg=uysum/(m_max-m_min+1);
        
        % wake center filtered
        % Should be calculated from mean air speed vs uyavg and lowpass filtered
        % Redo filter computation
        % yeff=ts*uyavg=grid.size/uxmean*uyavg
        % wc=wc+alpha*uyavg
        
        %Uncomment to reproduce bug
        %wc=p.wt.y;
        
        %Uncomment to reproduce meandering limits
        %wc=min(max(wc,p.wt.y-150),p.wt.y+150);
        
        %Notice here ts such that wake moves with uyavg speed
        wc=wc+p.ts*uyavg;
    end
end

== matlab function Wind Field/Wind Speed1/Wind speed ==
  data: ux input double; def input double; p parameter double; uavg output double; upoint output double
function [uavg, upoint] = windspeed(ux,def,p) %#eml
% ux    is the wind vector at the x position of the turbine
% def   is the vector deficits at each turbine
% p     is a structure containing parameters  

uavg = def(p.wt.num)*sum(ux)/(p.wind_grid);
upoint = def(p.wt.num)*ux(ceil(p.wind_grid/2));

== matlab function Wind Field/Wake2/Wake center and diameter ==
  data: uy input double; CT input double; p parameter double; w_c output double; w_d output double
function [w_c,w_d]=wake(uy,CT,p)
    % Todo: Change length(uy)*grid_size to farm size?
    %       Change such that parm is passed directly

    w_c=zeros(length(p.farm.x),1);
    w_d=zeros(length(p.farm.x),1);
    if CT < 1
        beta_w = (1+sqrt(1-CT))/(2*sqrt(1-CT));
    else
        beta_w = 1;
    end
    %beta_w = (1+sqrt(1-CT))/(2*sqrt(1-CT));
    %Find wake center and deficit for all other turbines
    for i=1:length(p.farm.x)
        delta_dist = p.farm.x(i)-p.wt.x;
        if(delta_dist>0) %Is turbine P downwind?
            w_d(i)= (beta_w^(p.k/2) +p.alpha*delta_dist/(2*p.wt.rotor.r))^(1/p.k)*2*p.wt.rotor.r;
            w_c(i)=wakecenter(uy,p.farm.x(i),p);%max(min(wakecenter(uy,p.farm.x(i),p),p.grid.ysize),1);
            
        else
            w_c(i)=p.wt.y;
            w_d(i)=0;
        end
    end
end


function wc=wakecenter(uy,px,p)
    
    %computer number of grid points to px
    it=ceil((px-p.wt.x)/p.grid.size);
    
    wc=p.wt.y; %wake center starts at turbine position
    
    for i=1:it %Compute the meandering for each grid point
        uysum=0;
        %compute the grid number for low meandering edge
        m_min=max(floor((wc-p.wt.meand.r(i))/p.grid.size)+1,1); 
        %compute the grid number for the high meandering edge
        m_max=min(ceil((wc+p.wt.meand.r(i))/p.grid.size)+1,length(uy)); 
        
        %average transversal velocity over the wake area
        for j=m_min:m_max
            uysum=uysum+uy(j);
        end
        uyavg=uysum/(m_max-m_min+1);
        
        % wake center filtered
        % Should be calculated from mean air speed vs uyavg and lowpass filtered
        % Redo filter computation
        % yeff=ts*uyavg=grid.size/uxmean*uyavg
        % wc=wc+alpha*uyavg
        
        %Uncomment to reproduce bug
        %wc=p.wt.y;
        
        %Uncomment to reproduce meandering limits
        %wc=min(max(wc,p.wt.y-150),p.wt.y+150);
        
        %Notice here ts such that wake moves with uyavg speed
        wc=wc+p.ts*uyavg;
    end
end

== matlab function Wind Field/Wind Speed2/Wind speed ==
  data: ux input double; def input double; p parameter double; uavg output double; upoint output double
function [uavg, upoint] = windspeed(ux,def,p) %#eml
% ux    is the wind vector at the x position of the turbine
% def   is the vector deficits at each turbine
% p     is a structure containing parameters  

uavg = def(p.wt.num)*sum(ux)/(p.wind_grid);
upoint = def(p.wt.num)*ux(ceil(p.wind_grid/2));

== matlab function Wind Field/Wake3/Wake center and diameter ==
  data: uy input double; CT input double; p parameter double; w_c output double; w_d output double
function [w_c,w_d]=wake(uy,CT,p)
    % Todo: Change length(uy)*grid_size to farm size?
    %       Change such that parm is passed directly

    w_c=zeros(length(p.farm.x),1);
    w_d=zeros(length(p.farm.x),1);
    if CT < 1
        beta_w = (1+sqrt(1-CT))/(2*sqrt(1-CT));
    else
        beta_w = 1;
    end
    %beta_w = (1+sqrt(1-CT))/(2*sqrt(1-CT));
    %Find wake center and deficit for all other turbines
    for i=1:length(p.farm.x)
        delta_dist = p.farm.x(i)-p.wt.x;
        if(delta_dist>0) %Is turbine P downwind?
            w_d(i)= (beta_w^(p.k/2) +p.alpha*delta_dist/(2*p.wt.rotor.r))^(1/p.k)*2*p.wt.rotor.r;
            w_c(i)=wakecenter(uy,p.farm.x(i),p);%max(min(wakecenter(uy,p.farm.x(i),p),p.grid.ysize),1);
            
        else
            w_c(i)=p.wt.y;
            w_d(i)=0;
        end
    end
end


function wc=wakecenter(uy,px,p)
    
    %computer number of grid points to px
    it=ceil((px-p.wt.x)/p.grid.size);
    
    wc=p.wt.y; %wake center starts at turbine position
    
    for i=1:it %Compute the meandering for each grid point
        uysum=0;
        %compute the grid number for low meandering edge
        m_min=max(floor((wc-p.wt.meand.r(i))/p.grid.size)+1,1); 
        %compute the grid number for the high meandering edge
        m_max=min(ceil((wc+p.wt.meand.r(i))/p.grid.size)+1,length(uy)); 
        
        %average transversal velocity over the wake area
        for j=m_min:m_max
            uysum=uysum+uy(j);
        end
        uyavg=uysum/(m_max-m_min+1);
        
        % wake center filtered
        % Should be calculated from mean air speed vs uyavg and lowpass filtered
        % Redo filter computation
        % yeff=ts*uyavg=grid.size/uxmean*uyavg
        % wc=wc+alpha*uyavg
        
        %Uncomment to reproduce bug
        %wc=p.wt.y;
        
        %Uncomment to reproduce meandering limits
        %wc=min(max(wc,p.wt.y-150),p.wt.y+150);
        
        %Notice here ts such that wake moves with uyavg speed
        wc=wc+p.ts*uyavg;
    end
end

== matlab function Wind Field/Wind Speed3/Wind speed ==
  data: ux input double; def input double; p parameter double; uavg output double; upoint output double
function [uavg, upoint] = windspeed(ux,def,p) %#eml
% ux    is the wind vector at the x position of the turbine
% def   is the vector deficits at each turbine
% p     is a structure containing parameters  

uavg = def(p.wt.num)*sum(ux)/(p.wind_grid);
upoint = def(p.wt.num)*ux(ceil(p.wind_grid/2));

== matlab function Wind Field/Wake4/Wake center and diameter ==
  data: uy input double; CT input double; p parameter double; w_c output double; w_d output double
function [w_c,w_d]=wake(uy,CT,p)
    % Todo: Change length(uy)*grid_size to farm size?
    %       Change such that parm is passed directly

    w_c=zeros(length(p.farm.x),1);
    w_d=zeros(length(p.farm.x),1);
    if CT < 1
        beta_w = (1+sqrt(1-CT))/(2*sqrt(1-CT));
    else
        beta_w = 1;
    end
    %beta_w = (1+sqrt(1-CT))/(2*sqrt(1-CT));
    %Find wake center and deficit for all other turbines
    for i=1:length(p.farm.x)
        delta_dist = p.farm.x(i)-p.wt.x;
        if(delta_dist>0) %Is turbine P downwind?
            w_d(i)= (beta_w^(p.k/2) +p.alpha*delta_dist/(2*p.wt.rotor.r))^(1/p.k)*2*p.wt.rotor.r;
            w_c(i)=wakecenter(uy,p.farm.x(i),p);%max(min(wakecenter(uy,p.farm.x(i),p),p.grid.ysize),1);
            
        else
            w_c(i)=p.wt.y;
            w_d(i)=0;
        end
    end
end


function wc=wakecenter(uy,px,p)
    
    %computer number of grid points to px
    it=ceil((px-p.wt.x)/p.grid.size);
    
    wc=p.wt.y; %wake center starts at turbine position
    
    for i=1:it %Compute the meandering for each grid point
        uysum=0;
        %compute the grid number for low meandering edge
        m_min=max(floor((wc-p.wt.meand.r(i))/p.grid.size)+1,1); 
        %compute the grid number for the high meandering edge
        m_max=min(ceil((wc+p.wt.meand.r(i))/p.grid.size)+1,length(uy)); 
        
        %average transversal velocity over the wake area
        for j=m_min:m_max
            uysum=uysum+uy(j);
        end
        uyavg=uysum/(m_max-m_min+1);
        
        % wake center filtered
        % Should be calculated from mean air speed vs uyavg and lowpass filtered
        % Redo filter computation
        % yeff=ts*uyavg=grid.size/uxmean*uyavg
        % wc=wc+alpha*uyavg
        
        %Uncomment to reproduce bug
        %wc=p.wt.y;
        
        %Uncomment to reproduce meandering limits
        %wc=min(max(wc,p.wt.y-150),p.wt.y+150);
        
        %Notice here ts such that wake moves with uyavg speed
        wc=wc+p.ts*uyavg;
    end
end

== matlab function Wind Field/Wind Speed4/Wind speed ==
  data: ux input double; def input double; p parameter double; uavg output double; upoint output double
function [uavg, upoint] = windspeed(ux,def,p) %#eml
% ux    is the wind vector at the x position of the turbine
% def   is the vector deficits at each turbine
% p     is a structure containing parameters  

uavg = def(p.wt.num)*sum(ux)/(p.wind_grid);
upoint = def(p.wt.num)*ux(ceil(p.wind_grid/2));

== matlab function Wind Field/Wake5/Wake center and diameter ==
  data: uy input double; CT input double; p parameter double; w_c output double; w_d output double
function [w_c,w_d]=wake(uy,CT,p)
    % Todo: Change length(uy)*grid_size to farm size?
    %       Change such that parm is passed directly

    w_c=zeros(length(p.farm.x),1);
    w_d=zeros(length(p.farm.x),1);
    if CT < 1
        beta_w = (1+sqrt(1-CT))/(2*sqrt(1-CT));
    else
        beta_w = 1;
    end
    %beta_w = (1+sqrt(1-CT))/(2*sqrt(1-CT));
    %Find wake center and deficit for all other turbines
    for i=1:length(p.farm.x)
        delta_dist = p.farm.x(i)-p.wt.x;
        if(delta_dist>0) %Is turbine P downwind?
            w_d(i)= (beta_w^(p.k/2) +p.alpha*delta_dist/(2*p.wt.rotor.r))^(1/p.k)*2*p.wt.rotor.r;
            w_c(i)=wakecenter(uy,p.farm.x(i),p);%max(min(wakecenter(uy,p.farm.x(i),p),p.grid.ysize),1);
            
        else
            w_c(i)=p.wt.y;
            w_d(i)=0;
        end
    end
end


function wc=wakecenter(uy,px,p)
    
    %computer number of grid points to px
    it=ceil((px-p.wt.x)/p.grid.size);
    
    wc=p.wt.y; %wake center starts at turbine position
    
    for i=1:it %Compute the meandering for each grid point
        uysum=0;
        %compute the grid number for low meandering edge
        m_min=max(floor((wc-p.wt.meand.r(i))/p.grid.size)+1,1); 
        %compute the grid number for the high meandering edge
        m_max=min(ceil((wc+p.wt.meand.r(i))/p.grid.size)+1,length(uy)); 
        
        %average transversal velocity over the wake area
        for j=m_min:m_max
            uysum=uysum+uy(j);
        end
        uyavg=uysum/(m_max-m_min+1);
        
        % wake center filtered
        % Should be calculated from mean air speed vs uyavg and lowpass filtered
        % Redo filter computation
        % yeff=ts*uyavg=grid.size/uxmean*uyavg
        % wc=wc+alpha*uyavg
        
        %Uncomment to reproduce bug
        %wc=p.wt.y;
        
        %Uncomment to reproduce meandering limits
        %wc=min(max(wc,p.wt.y-150),p.wt.y+150);
        
        %Notice here ts such that wake moves with uyavg speed
        wc=wc+p.ts*uyavg;
    end
end

== matlab function Wind Field/Wind Speed5/Wind speed ==
  data: ux input double; def input double; p parameter double; uavg output double; upoint output double
function [uavg, upoint] = windspeed(ux,def,p) %#eml
% ux    is the wind vector at the x position of the turbine
% def   is the vector deficits at each turbine
% p     is a structure containing parameters  

uavg = def(p.wt.num)*sum(ux)/(p.wind_grid);
upoint = def(p.wt.num)*ux(ceil(p.wind_grid/2));

== matlab function Wind Field/Wake6/Wake center and diameter ==
  data: uy input double; CT input double; p parameter double; w_c output double; w_d output double
function [w_c,w_d]=wake(uy,CT,p)
    % Todo: Change length(uy)*grid_size to farm size?
    %       Change such that parm is passed directly

    w_c=zeros(length(p.farm.x),1);
    w_d=zeros(length(p.farm.x),1);
    if CT < 1
        beta_w = (1+sqrt(1-CT))/(2*sqrt(1-CT));
    else
        beta_w = 1;
    end
    %beta_w = (1+sqrt(1-CT))/(2*sqrt(1-CT));
    %Find wake center and deficit for all other turbines
    for i=1:length(p.farm.x)
        delta_dist = p.farm.x(i)-p.wt.x;
        if(delta_dist>0) %Is turbine P downwind?
            w_d(i)= (beta_w^(p.k/2) +p.alpha*delta_dist/(2*p.wt.rotor.r))^(1/p.k)*2*p.wt.rotor.r;
            w_c(i)=wakecenter(uy,p.farm.x(i),p);%max(min(wakecenter(uy,p.farm.x(i),p),p.grid.ysize),1);
            
        else
            w_c(i)=p.wt.y;
            w_d(i)=0;
        end
    end
end


function wc=wakecenter(uy,px,p)
    
    %computer number of grid points to px
    it=ceil((px-p.wt.x)/p.grid.size);
    
    wc=p.wt.y; %wake center starts at turbine position
    
    for i=1:it %Compute the meandering for each grid point
        uysum=0;
        %compute the grid number for low meandering edge
        m_min=max(floor((wc-p.wt.meand.r(i))/p.grid.size)+1,1); 
        %compute the grid number for the high meandering edge
        m_max=min(ceil((wc+p.wt.meand.r(i))/p.grid.size)+1,length(uy)); 
        
        %average transversal velocity over the wake area
        for j=m_min:m_max
            uysum=uysum+uy(j);
        end
        uyavg=uysum/(m_max-m_min+1);
        
        % wake center filtered
        % Should be calculated from mean air speed vs uyavg and lowpass filtered
        % Redo filter computation
        % yeff=ts*uyavg=grid.size/uxmean*uyavg
        % wc=wc+alpha*uyavg
        
        %Uncomment to reproduce bug
        %wc=p.wt.y;
        
        %Uncomment to reproduce meandering limits
        %wc=min(max(wc,p.wt.y-150),p.wt.y+150);
        
        %Notice here ts such that wake moves with uyavg speed
        wc=wc+p.ts*uyavg;
    end
end

== matlab function Wind Field/Wind Speed6/Wind speed ==
  data: ux input double; def input double; p parameter double; uavg output double; upoint output double
function [uavg, upoint] = windspeed(ux,def,p) %#eml
% ux    is the wind vector at the x position of the turbine
% def   is the vector deficits at each turbine
% p     is a structure containing parameters  

uavg = def(p.wt.num)*sum(ux)/(p.wind_grid);
upoint = def(p.wt.num)*ux(ceil(p.wind_grid/2));
